simulink model "PrkgClimaActvMgr"  (R2022b)
settings: Solver=FixedStepDiscrete  StartTime=0.0  StopTime=10.0  FixedStep=0.2  RelTol=1e-3
(part 2 of 4)

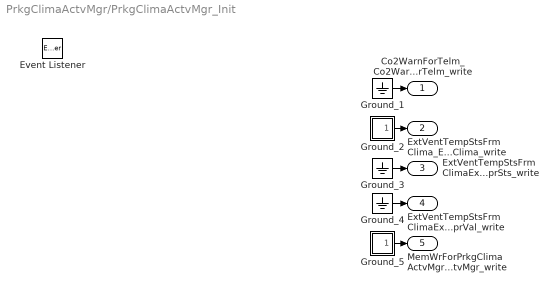
== system PrkgClimaActvMgr/PrkgClimaActvMgr_Init ==
  Event Listener : EventListener  EventName=reset
  Ground_1 : Ground
  Ground_2 : Subsystem
  Ground_3 : Ground
  Ground_4 : Ground
  Ground_5 : Subsystem
  Co2WarnForTelm_Co2WarnForTelm_write : Outport
  ExtVentTempStsFrmClima_ExtVentTempStsFrmClima_write : Outport  Port=2
  ExtVentTempStsFrmClimaExtVentTemprSts_ExtVentTempStsFrmClimaExtVentTemprSts_write : Outport  Port=3
  ExtVentTempStsFrmClimaExtVentTemprVal_ExtVentTempStsFrmClimaExtVentTemprVal_write : Outport  Port=4
  MemWrForPrkgClimaActvMgr_MemWrForPrkgClimaActvMgr_write : Outport  Port=5
  wires:
  Ground_1/1 -> Co2WarnForTelm_Co2WarnForTelm_write/1
  Ground_2/1(Out1) -> ExtVentTempStsFrmClima_ExtVentTempStsFrmClima_write/1
  Ground_3/1 -> ExtVentTempStsFrmClimaExtVentTemprSts_ExtVentTempStsFrmClimaExtVentTemprSts_write/1
  Ground_4/1 -> ExtVentTempStsFrmClimaExtVentTemprVal_ExtVentTempStsFrmClimaExtVentTemprVal_write/1
  Ground_5/1(Out1) -> MemWrForPrkgClimaActvMgr_MemWrForPrkgClimaActvMgr_write/1
